simulink model "AcasXuClosedLoop_all"  (Simulink 10.0)
settings: Solver=VariableStepAuto  StartTime=0.0  StopTime=20  RelTol=1e-3
libraries: neural x14040, simulink x2
(part 35 of 534)

== system AcasXuClosedLoop_all/networks/Networks_m_8/AcasXu_4_8/Process Output 1 ==
  a : Inport  PortDimensions=5
      mask Display: e = 0.06;c1 = [120 230 180]/255;c2 = ([120 230 180]+0)/(256+128);c3 = ([120 230 180]+128)/(256+128);patch([0 1 1 0],[0 0 1 1],c1);patch([0 1 1 1-e 1-e 0+e],[0 0 1 1-e 0+e 0+e],c2);patch([0 0 1 1-e 0+e 0+e],[0 1 1 1-e 1-e 0+e],c3);plot([0 1 1 0 0],[0 0 1 1 0]);
  y : Outport  PortDimensions=5
      mask Display: e = 0.06;c1 = [120 230 180]/255;c2 = ([120 230 180]+0)/(256+128);c3 = ([120 230 180]+128)/(256+128);patch([0 1 1 0],[0 0 1 1],c1);patch([0 1 1 1-e 1-e 0+e],[0 0 1 1-e 0+e 0+e],c2);patch([0 0 1 1-e 0+e 0+e],[0 1 1 1-e 1-e 0+e],c3);plot([0 1 1 0 0],[0 0 1 1 0]);
  wires:
  a/1 -> y/1

== system AcasXuClosedLoop_all/networks/Networks_m_8/AcasXu_5_8/Layer 1 ==
  "p{1}" : Inport  PortDimensions=5
      mask Display: e = 0.06;c1 = [120 230 180]/255;c2 = ([120 230 180]+0)/(256+128);c3 = ([120 230 180]+128)/(256+128);patch([0 1 1 0],[0 0 1 1],c1);patch([0 1 1 1-e 1-e 0+e],[0 0 1 1-e 0+e 0+e],c2);patch([0 0 1 1-e 0+e 0+e],[0 1 1 1-e 1-e 0+e],c3);plot([0 1 1 0 0],[0 0 1 1 0]);
  Delays 1 : Subsystem
      mask Display: e = 0.06;c1 = [120 150 230]/255;c2 = ([120 150 230]+0)/383;c3 = ([120 150 230]+128)/383;patch([0 1 1 0],[0 0 1 1],c1);patch([0 1 1 1-e 1-e 0+e],[0 0 1 1-e 0+e 0+e],c2);patch([0 0 1 1-e 0+e 0+e],[0 1 1 1-e 1-e 0+e],c3);plot([0 1 1 0 0],[0 0 1 1 0]);disp('0');
  "IW{1,1}" : Subsystem
      mask Display: e = 0.06;c1 = [120 150 230]/255;c2 = ([120 150 230]+0)/383;c3 = ([120 150 230]+128)/383;patch([0 1 1 0],[0 0 1 1],c1);patch([0 1 1 1-e 1-e 0+e],[0 0 1 1-e 0+e 0+e],c2);patch([0 0 1 1-e 0+e 0+e],[0 1 1 1-e 1-e 0+e],c3);plot([0 1 1 0 0],[0 0 1 1 0]);disp('W');
  "b{1}" : Constant  Value=[-0.319583000000000005957900839348440058529376983642578125;-0.3150749999999999939603867460391484200954437255859375;-0.09534789999999999932089878029728424735367298126220703125;-0.155059000000000002383870878475136123597621917724609375;0.034337600000000002842259760882370756007730960845947265625;-0.421516000000000001790567694115452468395233154296875;0.0857165000000000010249578963339445181190967559814453125;-0.46167700000000000404298816647497005760669708251953125;-0.176536999999999999477751089216326363384723663330078125;-0.05784999999999999864552790995730902068316936492919921875;-0.0216248999999999989840571146260117529891431331634521484375;0.298148000000000024112267738018999807536602020263671875;-0.2051790000000000002700062395888380706310272216796875;-0.27237699999999998023980651851161383092403411865234375;-0.05032319999999999848849796535432687960565090179443359375;0.1063159999999999938413708377993316389620304107666015625;-0.144828000000000012281731187613331712782382965087890625;-0.33846900000000001984545860977959819138050079345703125;-0.0590398000000000033882230354720377363264560699462890625;0.0077789299999999998724842242836530203931033611297607421875;-0.2140189999999999870272660018599708564579486846923828125;-0.422790999999999972391862002041307277977466583251953125;0.2399779999999999968718356058161589317023754119873046875;-0.15526899999999999035793507573544047772884368896484375;-0.1191190000000000026592061885821749456226825714111328125;-0.489229999999999998205879592205747030675411224365234375;-0.01832330000000000069793060220035840757191181182861328125;-0.036199299999999996757527043200752814300358295440673828125;-0.42294500000000001538325022920616902410984039306640625;-0.62282000000000004025224598080967552959918975830078125;-0.05856619999999999881534762380397296510636806488037109375;0.13910400000000000542144107384956441819667816162109375;-0.310653999999999985703880156506784260272979736328125;0.0285514000000000010837108987971078022383153438568115234375;-0.341386000000000022769341967432410456240177154541015625;-0.038599500000000001864730592160412925295531749725341796875;0.11351899999999999490984947669858229346573352813720703125;-0.337141999999999997239541471572010777890682220458984375;-0.386535999999999990706101016257889568805694580078125;-0.328332000000000012729373111142194829881191253662109375;-0.1999869999999999981010745386811322532594203948974609375;-0.10244399999999999339994616320836939848959445953369140625;0.182161999999999990595966892215074039995670318603515625;-0.2535279999999999755999624539981596171855926513671875;-0.04500769999999999770867731285761692561209201812744140625;-0.07817380000000000161985980184908839873969554901123046875;-0.3433370000000000032969182939268648624420166015625;0.11499099999999999599520350557213532738387584686279296875;0.378197999999999978637532649372587911784648895263671875;-0.2001010000000000010889067425523535348474979400634765625]
      mask Display: e = 0.06;c1 = [120 150 230]/255;c2 = ([120 150 230]+0)/383;c3 = ([120 150 230]+128)/383;patch([0 1 1 0],[0 0 1 1],c1);patch([0 1 1 1-e 1-e 0+e],[0 0 1 1-e 0+e 0+e],c2);patch([0 0 1 1-e 0+e 0+e],[0 1 1 1-e 1-e 0+e],c3);plot([0 1 1 0 0],[0 0 1 1 0]);disp('b');
  netsum : Sum  Inputs=2  SaturateOnIntegerOverflow=on  InputSameDT=on  OutDataTypeStr=Inherit: Same as first input
      mask Display: e = 0.06;c1 = [120 150 230]/255;c2 = ([120 150 230]+0)/383;c3 = ([120 150 230]+128)/383;patch([0 1 1 0],[0 0 1 1],c1);patch([0 1 1 1-e 1-e 0+e],[0 0 1 1-e 0+e 0+e],c2);patch([0 0 1 1-e 0+e 0+e],[0 1 1 1-e 1-e 0+e],c3);plot([0 1 1 0 0],[0 0 1 1 0]);disp('+');
  poslin : neural/Transfer Functions/poslin
      settings: SourceProductName=Deep Learning Toolbox  SourceProductBaseCode=NN
  "a{1}" : Outport  InitialOutput=[0;0;0;0;0;0;0;0;0;0;0;0;0;0;0;0;0;0;0;0;0;0;0;0;0;0;0;0;0;0;0;0;0;0;0;0;0;0;0;0;0;0;0;0;0;0;0;0;0;0]
      mask Display: e = 0.06;c1 = [120 230 180]/255;c2 = ([120 230 180]+0)/(256+128);c3 = ([120 230 180]+128)/(256+128);patch([0 1 1 0],[0 0 1 1],c1);patch([0 1 1 1-e 1-e 0+e],[0 0 1 1-e 0+e 0+e],c2);patch([0 0 1 1-e 0+e 0+e],[0 1 1 1-e 1-e 0+e],c3);plot([0 1 1 0 0],[0 0 1 1 0]);
  wires:
  poslin/1 -> "a{1}"/1
  netsum/1 -> poslin/1
  "b{1}"/1 -> netsum/2
  "IW{1,1}"/1("iz{1,1}") -> netsum/1
  Delays 1/1("pd{1,1}") -> "IW{1,1}"/1("pd{1,1}")
  "p{1}"/1 -> Delays 1/1("p{1}")

== system AcasXuClosedLoop_all/networks/Networks_m_8/AcasXu_5_8/Layer 2 ==
  "a{1} " : Inport  PortDimensions=50
      mask Display: e = 0.06;c1 = [120 230 180]/255;c2 = ([120 230 180]+0)/(256+128);c3 = ([120 230 180]+128)/(256+128);patch([0 1 1 0],[0 0 1 1],c1);patch([0 1 1 1-e 1-e 0+e],[0 0 1 1-e 0+e 0+e],c2);patch([0 0 1 1-e 0+e 0+e],[0 1 1 1-e 1-e 0+e],c3);plot([0 1 1 0 0],[0 0 1 1 0]);
  Delays 1 : Subsystem
      mask Display: e = 0.06;c1 = [120 150 230]/255;c2 = ([120 150 230]+0)/383;c3 = ([120 150 230]+128)/383;patch([0 1 1 0],[0 0 1 1],c1);patch([0 1 1 1-e 1-e 0+e],[0 0 1 1-e 0+e 0+e],c2);patch([0 0 1 1-e 0+e 0+e],[0 1 1 1-e 1-e 0+e],c3);plot([0 1 1 0 0],[0 0 1 1 0]);disp('0');
  "LW{2,1}" : Subsystem
      mask Display: e = 0.06;c1 = [120 150 230]/255;c2 = ([120 150 230]+0)/383;c3 = ([120 150 230]+128)/383;patch([0 1 1 0],[0 0 1 1],c1);patch([0 1 1 1-e 1-e 0+e],[0 0 1 1-e 0+e 0+e],c2);patch([0 0 1 1-e 0+e 0+e],[0 1 1 1-e 1-e 0+e],c3);plot([0 1 1 0 0],[0 0 1 1 0]);disp('W');
  "b{2}" : Constant  Value=[0.8293500000000000316191517413244582712650299072265625;-0.037375499999999999001243367047209176234900951385498046875;-1.9736100000000000864730509420041926205158233642578125;-1.100190000000000001278976924368180334568023681640625;-1.525160000000000071196382123162038624286651611328125;-0.92608100000000004303757350498926825821399688720703125;-2.50565000000000015489831639570184051990509033203125;-0.023995200000000001250288761411866289563477039337158203125;0.22440599999999999436539610542240552604198455810546875;0.22149499999999999744204615126363933086395263671875;0.244974999999999998312461002569762058556079864501953125;0.685756000000000032201796784647740423679351806640625;-0.1502779999999999949178430824758834205567836761474609375;0.7911420000000000118944853966240771114826202392578125;-1.4556899999999999284483465089579112827777862548828125;-0.75029800000000002047073621724848635494709014892578125;-0.0119462000000000005683897796870951424352824687957763671875;-0.93398700000000001164579543910804204642772674560546875;0.333909999999999984598986202399828471243381500244140625;-0.00553113000000000042122305643488289206288754940032958984375;0.54637299999999999755573298898525536060333251953125;-0.0108263000000000005063061081500563886947929859161376953125;1.1451299999999999812416717759333550930023193359375;1.1682799999999999851496568226139061152935028076171875;1.466369999999999951256768326857127249240875244140625;-0.0118300999999999996437072269372947630472481250762939453125;0.449658999999999975383246919591329060494899749755859375;0.82613000000000003097966327914036810398101806640625;0.0337432000000000009709566484161769039928913116455078125;-0.220237999999999989331200822562095709145069122314453125;-0.600424999999999986499688020558096468448638916015625;-0.76253300000000001634958834983990527689456939697265625;-0.1156770000000000020445867221496882848441600799560546875;0.0762764999999999970814457128653884865343570709228515625;0.54749499999999995392130358595750294625759124755859375;-1.4459299999999999375432935266871936619281768798828125;-0.437894999999999978701481495590996928513050079345703125;0.1760150000000000047872816821836750023066997528076171875;-0.40112599999999998257038669180474244058132171630859375;-0.01016309999999999956477036988644613302312791347503662109375;0.044338099999999998346300600360336829908192157745361328125;-0.005221739999999999888580237694668539916165173053741455078125;1.54872999999999994003019310184754431247711181640625;-1.30051000000000005485389920067973434925079345703125;-0.94384800000000002029310053330846130847930908203125;-0.85577000000000003065991904804832302033901214599609375;-0.030165100000000000246558329308754764497280120849609375;-0.289264000000000021106671965753776021301746368408203125;-0.00971820999999999969587438641838161856867372989654541015625;0.41300999999999998824051772317034192383289337158203125]
      mask Display: e = 0.06;c1 = [120 150 230]/255;c2 = ([120 150 230]+0)/383;c3 = ([120 150 230]+128)/383;patch([0 1 1 0],[0 0 1 1],c1);patch([0 1 1 1-e 1-e 0+e],[0 0 1 1-e 0+e 0+e],c2);patch([0 0 1 1-e 0+e 0+e],[0 1 1 1-e 1-e 0+e],c3);plot([0 1 1 0 0],[0 0 1 1 0]);disp('b');
  netsum : Sum  Inputs=2  SaturateOnIntegerOverflow=on  InputSameDT=on  OutDataTypeStr=Inherit: Same as first input
      mask Display: e = 0.06;c1 = [120 150 230]/255;c2 = ([120 150 230]+0)/383;c3 = ([120 150 230]+128)/383;patch([0 1 1 0],[0 0 1 1],c1);patch([0 1 1 1-e 1-e 0+e],[0 0 1 1-e 0+e 0+e],c2);patch([0 0 1 1-e 0+e 0+e],[0 1 1 1-e 1-e 0+e],c3);plot([0 1 1 0 0],[0 0 1 1 0]);disp('+');
  poslin : neural/Transfer Functions/poslin
      settings: SourceProductName=Deep Learning Toolbox  SourceProductBaseCode=NN
  "a{2}" : Outport  InitialOutput=[0;0;0;0;0;0;0;0;0;0;0;0;0;0;0;0;0;0;0;0;0;0;0;0;0;0;0;0;0;0;0;0;0;0;0;0;0;0;0;0;0;0;0;0;0;0;0;0;0;0]
      mask Display: e = 0.06;c1 = [120 230 180]/255;c2 = ([120 230 180]+0)/(256+128);c3 = ([120 230 180]+128)/(256+128);patch([0 1 1 0],[0 0 1 1],c1);patch([0 1 1 1-e 1-e 0+e],[0 0 1 1-e 0+e 0+e],c2);patch([0 0 1 1-e 0+e 0+e],[0 1 1 1-e 1-e 0+e],c3);plot([0 1 1 0 0],[0 0 1 1 0]);
  wires:
  poslin/1 -> "a{2}"/1
  netsum/1 -> poslin/1
  "b{2}"/1 -> netsum/2
  "LW{2,1}"/1("lz{2,1}") -> netsum/1
  Delays 1/1("pd{2,1}") -> "LW{2,1}"/1("ad{2,1}")
  "a{1} "/1 -> Delays 1/1("p{2}")

== system AcasXuClosedLoop_all/networks/Networks_m_8/AcasXu_5_8/Layer 3 ==
  "a{2} " : Inport  PortDimensions=50
      mask Display: e = 0.06;c1 = [120 230 180]/255;c2 = ([120 230 180]+0)/(256+128);c3 = ([120 230 180]+128)/(256+128);patch([0 1 1 0],[0 0 1 1],c1);patch([0 1 1 1-e 1-e 0+e],[0 0 1 1-e 0+e 0+e],c2);patch([0 0 1 1-e 0+e 0+e],[0 1 1 1-e 1-e 0+e],c3);plot([0 1 1 0 0],[0 0 1 1 0]);
  Delays 1 : Subsystem
      mask Display: e = 0.06;c1 = [120 150 230]/255;c2 = ([120 150 230]+0)/383;c3 = ([120 150 230]+128)/383;patch([0 1 1 0],[0 0 1 1],c1);patch([0 1 1 1-e 1-e 0+e],[0 0 1 1-e 0+e 0+e],c2);patch([0 0 1 1-e 0+e 0+e],[0 1 1 1-e 1-e 0+e],c3);plot([0 1 1 0 0],[0 0 1 1 0]);disp('0');
  "LW{3,2}" : Subsystem
      mask Display: e = 0.06;c1 = [120 150 230]/255;c2 = ([120 150 230]+0)/383;c3 = ([120 150 230]+128)/383;patch([0 1 1 0],[0 0 1 1],c1);patch([0 1 1 1-e 1-e 0+e],[0 0 1 1-e 0+e 0+e],c2);patch([0 0 1 1-e 0+e 0+e],[0 1 1 1-e 1-e 0+e],c3);plot([0 1 1 0 0],[0 0 1 1 0]);disp('W');
  "b{3}" : Constant  Value=[0.6144659999999999566711039733490906655788421630859375;-1.150679999999999925108795650885440409183502197265625;1.506089999999999928803617876837961375713348388671875;-0.0218403999999999993975041689964200486429035663604736328125;1.099439999999999972857267493964172899723052978515625;0.65309399999999995234389871257008053362369537353515625;-0.67926399999999997891819702999782748520374298095703125;-0.586089999999999999857891452847979962825775146484375;0.3059439999999999937330130705959163606166839599609375;-0.9985239999999999671587147531681694090366363525390625;-1.3036700000000001065103560904390178620815277099609375;1.218690000000000051016968427575193345546722412109375;-1.231030000000000068638428274425677955150604248046875;-0.269017000000000006121325668573263101279735565185546875;-0.1322319999999999884376933323437697254121303558349609375;-0.64689399999999996904165300293243490159511566162109375;-1.992599999999999926814098216709680855274200439453125;-0.93583600000000000118660636871936731040477752685546875;-2.670059999999999877928757996414788067340850830078125;0.6867689999999999628244040650315582752227783203125;-1.8478300000000000835598257253877818584442138671875;-0.878910999999999997811528373858891427516937255859375;-1.1449800000000001087840928448713384568691253662109375;-0.644249999999999989341858963598497211933135986328125;-0.55706800000000000760991269999067299067974090576171875;-0.145936000000000010157208407690632157027721405029296875;-2.324780000000000068638428274425677955150604248046875;1.6987200000000000077449158197850920259952545166015625;-0.03332290000000000251834109121773508377373218536376953125;0.91283700000000000951416723182774148881435394287109375;-0.149413999999999991263877063829568214714527130126953125;-2.640660000000000007247535904753021895885467529296875;-0.0249848000000000014519940805257647298276424407958984375;-0.76798299999999997123012462907354347407817840576171875;1.3071900000000000741096073397784493863582611083984375;-1.425289999999999945856643535080365836620330810546875;-0.12238300000000000566924285294589935801923274993896484375;-0.01368040000000000054602988797114448971115052700042724609375;-0.7686349999999999571542730336659587919712066650390625;-0.1422120000000000050732751333271153271198272705078125;-0.176196999999999992514432278767344541847705841064453125;-1.058829999999999937898564894567243754863739013671875;-1.5299400000000000776623210185789503157138824462890625;-0.736450000000000049027448767446912825107574462890625;-0.84162999999999998923527755323448218405246734619140625;-0.043412899999999997324717782021252787671983242034912109375;-1.323560000000000069775296651641838252544403076171875;1.27156000000000002359001882723532617092132568359375;-1.148660000000000014352963262354023754596710205078125;-1.66657000000000010686562745831906795501708984375]
      mask Display: e = 0.06;c1 = [120 150 230]/255;c2 = ([120 150 230]+0)/383;c3 = ([120 150 230]+128)/383;patch([0 1 1 0],[0 0 1 1],c1);patch([0 1 1 1-e 1-e 0+e],[0 0 1 1-e 0+e 0+e],c2);patch([0 0 1 1-e 0+e 0+e],[0 1 1 1-e 1-e 0+e],c3);plot([0 1 1 0 0],[0 0 1 1 0]);disp('b');
  netsum : Sum  Inputs=2  SaturateOnIntegerOverflow=on  InputSameDT=on  OutDataTypeStr=Inherit: Same as first input
      mask Display: e = 0.06;c1 = [120 150 230]/255;c2 = ([120 150 230]+0)/383;c3 = ([120 150 230]+128)/383;patch([0 1 1 0],[0 0 1 1],c1);patch([0 1 1 1-e 1-e 0+e],[0 0 1 1-e 0+e 0+e],c2);patch([0 0 1 1-e 0+e 0+e],[0 1 1 1-e 1-e 0+e],c3);plot([0 1 1 0 0],[0 0 1 1 0]);disp('+');
  poslin : neural/Transfer Functions/poslin
      settings: SourceProductName=Deep Learning Toolbox  SourceProductBaseCode=NN
  "a{3}" : Outport  InitialOutput=[0;0;0;0;0;0;0;0;0;0;0;0;0;0;0;0;0;0;0;0;0;0;0;0;0;0;0;0;0;0;0;0;0;0;0;0;0;0;0;0;0;0;0;0;0;0;0;0;0;0]
      mask Display: e = 0.06;c1 = [120 230 180]/255;c2 = ([120 230 180]+0)/(256+128);c3 = ([120 230 180]+128)/(256+128);patch([0 1 1 0],[0 0 1 1],c1);patch([0 1 1 1-e 1-e 0+e],[0 0 1 1-e 0+e 0+e],c2);patch([0 0 1 1-e 0+e 0+e],[0 1 1 1-e 1-e 0+e],c3);plot([0 1 1 0 0],[0 0 1 1 0]);
  wires:
  poslin/1 -> "a{3}"/1
  netsum/1 -> poslin/1
  "b{3}"/1 -> netsum/2
  "LW{3,2}"/1("lz{3,2}") -> netsum/1
  Delays 1/1("pd{3,2}") -> "LW{3,2}"/1("ad{3,2}")
  "a{2} "/1 -> Delays 1/1("p{3}")

== system AcasXuClosedLoop_all/networks/Networks_m_8/AcasXu_5_8/Layer 4 ==
  "a{3} " : Inport  PortDimensions=50
      mask Display: e = 0.06;c1 = [120 230 180]/255;c2 = ([120 230 180]+0)/(256+128);c3 = ([120 230 180]+128)/(256+128);patch([0 1 1 0],[0 0 1 1],c1);patch([0 1 1 1-e 1-e 0+e],[0 0 1 1-e 0+e 0+e],c2);patch([0 0 1 1-e 0+e 0+e],[0 1 1 1-e 1-e 0+e],c3);plot([0 1 1 0 0],[0 0 1 1 0]);
  Delays 1 : Subsystem
      mask Display: e = 0.06;c1 = [120 150 230]/255;c2 = ([120 150 230]+0)/383;c3 = ([120 150 230]+128)/383;patch([0 1 1 0],[0 0 1 1],c1);patch([0 1 1 1-e 1-e 0+e],[0 0 1 1-e 0+e 0+e],c2);patch([0 0 1 1-e 0+e 0+e],[0 1 1 1-e 1-e 0+e],c3);plot([0 1 1 0 0],[0 0 1 1 0]);disp('0');
  "LW{4,3}" : Subsystem
      mask Display: e = 0.06;c1 = [120 150 230]/255;c2 = ([120 150 230]+0)/383;c3 = ([120 150 230]+128)/383;patch([0 1 1 0],[0 0 1 1],c1);patch([0 1 1 1-e 1-e 0+e],[0 0 1 1-e 0+e 0+e],c2);patch([0 0 1 1-e 0+e 0+e],[0 1 1 1-e 1-e 0+e],c3);plot([0 1 1 0 0],[0 0 1 1 0]);disp('W');
  "b{4}" : Constant  Value=[0.702555999999999958305352265597321093082427978515625;1.3879099999999999770494696349487639963626861572265625;0.3384570000000000078443918027915060520172119140625;1.8266199999999999104005610206513665616512298583984375;0.90297499999999997211119762141606770455837249755859375;-1.5656499999999999861444166526780463755130767822265625;2.740489999999999870539113544509746134281158447265625;-2.53134000000000014551915228366851806640625;-1.0149699999999999278088580467738211154937744140625;-0.41894500000000001183053655040566809475421905517578125;1.289029999999999898108171692001633346080780029296875;-1.0280400000000000648725517748971469700336456298828125;4.997939999999999827196006663143634796142578125;0.81166700000000002734878989940625615417957305908203125;-1.834379999999999899529257163521833717823028564453125;2.332240000000000090807361630140803754329681396484375;0.12376600000000000101341157687784289009869098663330078125;-0.2025119999999999975681674868610571138560771942138671875;1.195019999999999971151964928139932453632354736328125;0.8384679999999999910897940935683436691761016845703125;0.641885999999999956600049699773080646991729736328125;0.007645029999999999707760434120018544490449130535125732421875;-2.253229999999999844106923774234019219875335693359375;0.70936600000000005206146624914254061877727508544921875;-0.039787999999999996980637462229424272663891315460205078125;0.768780000000000018900436771218664944171905517578125;-0.97767099999999995674215824692510068416595458984375;0.211146000000000000351718654201249592006206512451171875;-0.83741500000000002046363078989088535308837890625;-1.753509999999999902087211012258194386959075927734375;0.397567999999999976967757220336352474987506866455078125;-0.417839000000000015955237131493049673736095428466796875;1.2331399999999999028688080215943045914173126220703125;1.6854999999999999982236431605997495353221893310546875;0.06742479999999999307203069065508316271007061004638671875;2.401850000000000040500935938325710594654083251953125;-0.0266778000000000015123902130653732456266880035400390625;-2.4404599999999998516386767732910811901092529296875;0.26406600000000002292921408297843299806118011474609375;-0.6424499999999999655386773156351409852504730224609375;1.0294600000000000417088585891178809106349945068359375;0.84932700000000005413625103756203316152095794677734375;0.93856700000000004013855914308805949985980987548828125;1.319269999999999942730255497735925018787384033203125;-1.5707999999999999740651901447563432157039642333984375;0.8400069999999999481588019989430904388427734375;0.8738289999999999668744976588641293346881866455078125;-0.1663550000000000028688162956314045004546642303466796875;1.4832799999999999318589516406063921749591827392578125;0.98826300000000000256505927609396167099475860595703125]
      mask Display: e = 0.06;c1 = [120 150 230]/255;c2 = ([120 150 230]+0)/383;c3 = ([120 150 230]+128)/383;patch([0 1 1 0],[0 0 1 1],c1);patch([0 1 1 1-e 1-e 0+e],[0 0 1 1-e 0+e 0+e],c2);patch([0 0 1 1-e 0+e 0+e],[0 1 1 1-e 1-e 0+e],c3);plot([0 1 1 0 0],[0 0 1 1 0]);disp('b');
  netsum : Sum  Inputs=2  SaturateOnIntegerOverflow=on  InputSameDT=on  OutDataTypeStr=Inherit: Same as first input
      mask Display: e = 0.06;c1 = [120 150 230]/255;c2 = ([120 150 230]+0)/383;c3 = ([120 150 230]+128)/383;patch([0 1 1 0],[0 0 1 1],c1);patch([0 1 1 1-e 1-e 0+e],[0 0 1 1-e 0+e 0+e],c2);patch([0 0 1 1-e 0+e 0+e],[0 1 1 1-e 1-e 0+e],c3);plot([0 1 1 0 0],[0 0 1 1 0]);disp('+');
  poslin : neural/Transfer Functions/poslin
      settings: SourceProductName=Deep Learning Toolbox  SourceProductBaseCode=NN
  "a{4}" : Outport  InitialOutput=[0;0;0;0;0;0;0;0;0;0;0;0;0;0;0;0;0;0;0;0;0;0;0;0;0;0;0;0;0;0;0;0;0;0;0;0;0;0;0;0;0;0;0;0;0;0;0;0;0;0]
      mask Display: e = 0.06;c1 = [120 230 180]/255;c2 = ([120 230 180]+0)/(256+128);c3 = ([120 230 180]+128)/(256+128);patch([0 1 1 0],[0 0 1 1],c1);patch([0 1 1 1-e 1-e 0+e],[0 0 1 1-e 0+e 0+e],c2);patch([0 0 1 1-e 0+e 0+e],[0 1 1 1-e 1-e 0+e],c3);plot([0 1 1 0 0],[0 0 1 1 0]);
  wires:
  poslin/1 -> "a{4}"/1
  netsum/1 -> poslin/1
  "b{4}"/1 -> netsum/2
  "LW{4,3}"/1("lz{4,3}") -> netsum/1
  Delays 1/1("pd{4,3}") -> "LW{4,3}"/1("ad{4,3}")
  "a{3} "/1 -> Delays 1/1("p{4}")

== system AcasXuClosedLoop_all/networks/Networks_m_8/AcasXu_5_8/Layer 5 ==
  "a{4} " : Inport  PortDimensions=50
      mask Display: e = 0.06;c1 = [120 230 180]/255;c2 = ([120 230 180]+0)/(256+128);c3 = ([120 230 180]+128)/(256+128);patch([0 1 1 0],[0 0 1 1],c1);patch([0 1 1 1-e 1-e 0+e],[0 0 1 1-e 0+e 0+e],c2);patch([0 0 1 1-e 0+e 0+e],[0 1 1 1-e 1-e 0+e],c3);plot([0 1 1 0 0],[0 0 1 1 0]);
  Delays 1 : Subsystem
      mask Display: e = 0.06;c1 = [120 150 230]/255;c2 = ([120 150 230]+0)/383;c3 = ([120 150 230]+128)/383;patch([0 1 1 0],[0 0 1 1],c1);patch([0 1 1 1-e 1-e 0+e],[0 0 1 1-e 0+e 0+e],c2);patch([0 0 1 1-e 0+e 0+e],[0 1 1 1-e 1-e 0+e],c3);plot([0 1 1 0 0],[0 0 1 1 0]);disp('0');
  "LW{5,4}" : Subsystem
      mask Display: e = 0.06;c1 = [120 150 230]/255;c2 = ([120 150 230]+0)/383;c3 = ([120 150 230]+128)/383;patch([0 1 1 0],[0 0 1 1],c1);patch([0 1 1 1-e 1-e 0+e],[0 0 1 1-e 0+e 0+e],c2);patch([0 0 1 1-e 0+e 0+e],[0 1 1 1-e 1-e 0+e],c3);plot([0 1 1 0 0],[0 0 1 1 0]);disp('W');
  "b{5}" : Constant  Value=[-3.1370599999999999596411726088263094425201416015625;-1.6457100000000000061817218011128716170787811279296875;-1.575390000000000068069994085817597806453704833984375;-0.271681000000000005822897719554021023213863372802734375;0.4875220000000000108997255665599368512630462646484375;1.5458700000000000773781039242749102413654327392578125;-1.087099999999999955235807647113688290119171142578125;-0.096647800000000005926636958974995650351047515869140625;1.4024900000000000144240175359300337731838226318359375;0.8425219999999999931361571725574322044849395751953125;0.6656800000000000494537744089029729366302490234375;-1.018520000000000091944230007356964051723480224609375;1.1327899999999999636202119290828704833984375;2.48627000000000020207835405017249286174774169921875;0.6509570000000000078443918027915060520172119140625;-0.042899100000000002508304675075123668648302555084228515625;-1.2160800000000000498090457767830230295658111572265625;-2.937650000000000094502183856093324720859527587890625;1.667829999999999923687710179365240037441253662109375;-3.67757999999999984908072292455472052097320556640625;0.6318070000000000074891204349114559590816497802734375;-0.479887000000000008004263918337528593838214874267578125;2.622430000000000038795633372501470148563385009765625;4.37605000000000021742607714259065687656402587890625;0.48666500000000001424638185199000872671604156494140625;-2.939290000000000180335746335913427174091339111328125;-0.4961160000000000014352963262354023754596710205078125;2.286119999999999929940486254054121673107147216796875;-1.5830999999999999516830939683131873607635498046875;-1.6355999999999999428013097713119350373744964599609375;-2.411880000000000023874235921539366245269775390625;-1.1924799999999999844391140868538059294223785400390625;-2.653000000000000024868995751603506505489349365234375;0.68499900000000002453504066579625941812992095947265625;0.9526369999999999560458263658802025020122528076171875;-1.234669999999999934203742668614722788333892822265625;2.191619999999999901518776823650114238262176513671875;-4.706559999999999632791514159180223941802978515625;-0.475185000000000024034108037085388787090778350830078125;0.324878999999999973358200122675043530762195587158203125;1.14918999999999993377741702715866267681121826171875;-0.56789500000000003865352482534945011138916015625;-3.545409999999999950404117043945007026195526123046875;1.9624900000000000677147227179375477135181427001953125;-0.057324199999999998766941899930316139943897724151611328125;-1.4606600000000000694200252837617881596088409423828125;1.7740199999999999302957576219341717660427093505859375;0.11874200000000000032596148002994596026837825775146484375;-1.5381000000000000227373675443232059478759765625;-4.32064000000000003609557097661308944225311279296875]
      mask Display: e = 0.06;c1 = [120 150 230]/255;c2 = ([120 150 230]+0)/383;c3 = ([120 150 230]+128)/383;patch([0 1 1 0],[0 0 1 1],c1);patch([0 1 1 1-e 1-e 0+e],[0 0 1 1-e 0+e 0+e],c2);patch([0 0 1 1-e 0+e 0+e],[0 1 1 1-e 1-e 0+e],c3);plot([0 1 1 0 0],[0 0 1 1 0]);disp('b');
  netsum : Sum  Inputs=2  SaturateOnIntegerOverflow=on  InputSameDT=on  OutDataTypeStr=Inherit: Same as first input
      mask Display: e = 0.06;c1 = [120 150 230]/255;c2 = ([120 150 230]+0)/383;c3 = ([120 150 230]+128)/383;patch([0 1 1 0],[0 0 1 1],c1);patch([0 1 1 1-e 1-e 0+e],[0 0 1 1-e 0+e 0+e],c2);patch([0 0 1 1-e 0+e 0+e],[0 1 1 1-e 1-e 0+e],c3);plot([0 1 1 0 0],[0 0 1 1 0]);disp('+');
  poslin : neural/Transfer Functions/poslin
      settings: SourceProductName=Deep Learning Toolbox  SourceProductBaseCode=NN
  "a{5}" : Outport  InitialOutput=[0;0;0;0;0;0;0;0;0;0;0;0;0;0;0;0;0;0;0;0;0;0;0;0;0;0;0;0;0;0;0;0;0;0;0;0;0;0;0;0;0;0;0;0;0;0;0;0;0;0]
      mask Display: e = 0.06;c1 = [120 230 180]/255;c2 = ([120 230 180]+0)/(256+128);c3 = ([120 230 180]+128)/(256+128);patch([0 1 1 0],[0 0 1 1],c1);patch([0 1 1 1-e 1-e 0+e],[0 0 1 1-e 0+e 0+e],c2);patch([0 0 1 1-e 0+e 0+e],[0 1 1 1-e 1-e 0+e],c3);plot([0 1 1 0 0],[0 0 1 1 0]);
  wires:
  poslin/1 -> "a{5}"/1
  netsum/1 -> poslin/1
  "b{5}"/1 -> netsum/2
  "LW{5,4}"/1("lz{5,4}") -> netsum/1
  Delays 1/1("pd{5,4}") -> "LW{5,4}"/1("ad{5,4}")
  "a{4} "/1 -> Delays 1/1("p{5}")

== system AcasXuClosedLoop_all/networks/Networks_m_8/AcasXu_5_8/Layer 6 ==
  "a{5} " : Inport  PortDimensions=50
      mask Display: e = 0.06;c1 = [120 230 180]/255;c2 = ([120 230 180]+0)/(256+128);c3 = ([120 230 180]+128)/(256+128);patch([0 1 1 0],[0 0 1 1],c1);patch([0 1 1 1-e 1-e 0+e],[0 0 1 1-e 0+e 0+e],c2);patch([0 0 1 1-e 0+e 0+e],[0 1 1 1-e 1-e 0+e],c3);plot([0 1 1 0 0],[0 0 1 1 0]);
  Delays 1 : Subsystem
      mask Display: e = 0.06;c1 = [120 150 230]/255;c2 = ([120 150 230]+0)/383;c3 = ([120 150 230]+128)/383;patch([0 1 1 0],[0 0 1 1],c1);patch([0 1 1 1-e 1-e 0+e],[0 0 1 1-e 0+e 0+e],c2);patch([0 0 1 1-e 0+e 0+e],[0 1 1 1-e 1-e 0+e],c3);plot([0 1 1 0 0],[0 0 1 1 0]);disp('0');
  "LW{6,5}" : Subsystem
      mask Display: e = 0.06;c1 = [120 150 230]/255;c2 = ([120 150 230]+0)/383;c3 = ([120 150 230]+128)/383;patch([0 1 1 0],[0 0 1 1],c1);patch([0 1 1 1-e 1-e 0+e],[0 0 1 1-e 0+e 0+e],c2);patch([0 0 1 1-e 0+e 0+e],[0 1 1 1-e 1-e 0+e],c3);plot([0 1 1 0 0],[0 0 1 1 0]);disp('W');
  "b{6}" : Constant  Value=[0.5970990000000000463842297904193401336669921875;3.27104999999999979110043568653054535388946533203125;-0.73021999999999998021138480908120982348918914794921875;5.6033600000000003404920789762400090694427490234375;0.058062099999999998434230263910649227909743785858154296875;-3.54084999999999983089082888909615576267242431640625;3.0053000000000000824229573481716215610504150390625;-0.033653700000000001668620797090625273995101451873779296875;-0.4597029999999999727577915109577588737010955810546875;-3.43447000000000013386625141720287501811981201171875;1.75800000000000000710542735760100185871124267578125;0.76190199999999996816058001058991067111492156982421875;-3.917429999999999967741359796491451561450958251953125;1.390589999999999992752464095246978104114532470703125;1.1082199999999999828759200681815855205059051513671875;-1.5958600000000000562749846721999347209930419921875;-0.19688600000000000544986278327996842563152313232421875;-2.475429999999999797211103214067406952381134033203125;-0.1586950000000000027267077484793844632804393768310546875;-2.147619999999999862438926356844604015350341796875;2.91274999999999995026200849679298698902130126953125;-2.2351299999999998391331246239133179187774658203125;-4.30694999999999961204366627498529851436614990234375;-0.029325400000000001465849663873086683452129364013671875;-0.014737699999999999411759432632607058621942996978759765625;-4.529379999999999739657141617499291896820068359375;0.06399839999999999695656782705555087886750698089599609375;4.03953999999999968650854498264379799365997314453125;0.59581600000000001227107304657693020999431610107421875;2.958439999999999958646412778762169182300567626953125;0.3424490000000000033963942769332788884639739990234375;1.580410000000000092512664195965044200420379638671875;0.45372299999999998743049900440382771193981170654296875;1.2287500000000000088817841970012523233890533447265625;0.70787400000000000321875859299325384199619293212890625;0.439097999999999988318677424103952944278717041015625;7.138700000000000045474735088646411895751953125;-1.1250199999999999089794755491311661899089813232421875;-2.954860000000000042064129956997931003570556640625;-1.0480899999999999661071115042432211339473724365234375;-2.107110000000000038511416278197430074214935302734375;1.720429999999999903792513578082434833049774169921875;-1.3264299999999999979394260662957094609737396240234375;0.85650400000000004308020606913487426936626434326171875;0.489951000000000025380586521350778639316558837890625;-1.5742700000000000581934500587522052228450775146484375;1.25326000000000004064304448547773063182830810546875;-3.311179999999999790105675856466405093669891357421875;1.8854699999999999793232063893810845911502838134765625;-0.8230049999999999865707422941341064870357513427734375]
      mask Display: e = 0.06;c1 = [120 150 230]/255;c2 = ([120 150 230]+0)/383;c3 = ([120 150 230]+128)/383;patch([0 1 1 0],[0 0 1 1],c1);patch([0 1 1 1-e 1-e 0+e],[0 0 1 1-e 0+e 0+e],c2);patch([0 0 1 1-e 0+e 0+e],[0 1 1 1-e 1-e 0+e],c3);plot([0 1 1 0 0],[0 0 1 1 0]);disp('b');
  netsum : Sum  Inputs=2  SaturateOnIntegerOverflow=on  InputSameDT=on  OutDataTypeStr=Inherit: Same as first input
      mask Display: e = 0.06;c1 = [120 150 230]/255;c2 = ([120 150 230]+0)/383;c3 = ([120 150 230]+128)/383;patch([0 1 1 0],[0 0 1 1],c1);patch([0 1 1 1-e 1-e 0+e],[0 0 1 1-e 0+e 0+e],c2);patch([0 0 1 1-e 0+e 0+e],[0 1 1 1-e 1-e 0+e],c3);plot([0 1 1 0 0],[0 0 1 1 0]);disp('+');
  poslin : neural/Transfer Functions/poslin
      settings: SourceProductName=Deep Learning Toolbox  SourceProductBaseCode=NN
  "a{6}" : Outport  InitialOutput=[0;0;0;0;0;0;0;0;0;0;0;0;0;0;0;0;0;0;0;0;0;0;0;0;0;0;0;0;0;0;0;0;0;0;0;0;0;0;0;0;0;0;0;0;0;0;0;0;0;0]
      mask Display: e = 0.06;c1 = [120 230 180]/255;c2 = ([120 230 180]+0)/(256+128);c3 = ([120 230 180]+128)/(256+128);patch([0 1 1 0],[0 0 1 1],c1);patch([0 1 1 1-e 1-e 0+e],[0 0 1 1-e 0+e 0+e],c2);patch([0 0 1 1-e 0+e 0+e],[0 1 1 1-e 1-e 0+e],c3);plot([0 1 1 0 0],[0 0 1 1 0]);
  wires:
  poslin/1 -> "a{6}"/1
  netsum/1 -> poslin/1
  "b{6}"/1 -> netsum/2
  "LW{6,5}"/1("lz{6,5}") -> netsum/1
  Delays 1/1("pd{6,5}") -> "LW{6,5}"/1("ad{6,5}")
  "a{5} "/1 -> Delays 1/1("p{6}")

== system AcasXuClosedLoop_all/networks/Networks_m_8/AcasXu_5_8/Layer 7 ==
  "a{6} " : Inport  PortDimensions=50
      mask Display: e = 0.06;c1 = [120 230 180]/255;c2 = ([120 230 180]+0)/(256+128);c3 = ([120 230 180]+128)/(256+128);patch([0 1 1 0],[0 0 1 1],c1);patch([0 1 1 1-e 1-e 0+e],[0 0 1 1-e 0+e 0+e],c2);patch([0 0 1 1-e 0+e 0+e],[0 1 1 1-e 1-e 0+e],c3);plot([0 1 1 0 0],[0 0 1 1 0]);
  Delays 1 : Subsystem
      mask Display: e = 0.06;c1 = [120 150 230]/255;c2 = ([120 150 230]+0)/383;c3 = ([120 150 230]+128)/383;patch([0 1 1 0],[0 0 1 1],c1);patch([0 1 1 1-e 1-e 0+e],[0 0 1 1-e 0+e 0+e],c2);patch([0 0 1 1-e 0+e 0+e],[0 1 1 1-e 1-e 0+e],c3);plot([0 1 1 0 0],[0 0 1 1 0]);disp('0');
  "LW{7,6}" : Subsystem
      mask Display: e = 0.06;c1 = [120 150 230]/255;c2 = ([120 150 230]+0)/383;c3 = ([120 150 230]+128)/383;patch([0 1 1 0],[0 0 1 1],c1);patch([0 1 1 1-e 1-e 0+e],[0 0 1 1-e 0+e 0+e],c2);patch([0 0 1 1-e 0+e 0+e],[0 1 1 1-e 1-e 0+e],c3);plot([0 1 1 0 0],[0 0 1 1 0]);disp('W');
  "b{7}" : Constant  Value=[-0.0200872999999999989062526850602807826362550258636474609375;0.018134799999999999531308247924243914894759654998779296875;-0.0185214999999999997692956554828924708999693393707275390625;0.0184039000000000008139267038131947629153728485107421875;-0.018308600000000001262190352235847967676818370819091796875]
      mask Display: e = 0.06;c1 = [120 150 230]/255;c2 = ([120 150 230]+0)/383;c3 = ([120 150 230]+128)/383;patch([0 1 1 0],[0 0 1 1],c1);patch([0 1 1 1-e 1-e 0+e],[0 0 1 1-e 0+e 0+e],c2);patch([0 0 1 1-e 0+e 0+e],[0 1 1 1-e 1-e 0+e],c3);plot([0 1 1 0 0],[0 0 1 1 0]);disp('b');
  netsum : Sum  Inputs=2  SaturateOnIntegerOverflow=on  InputSameDT=on  OutDataTypeStr=Inherit: Same as first input
      mask Display: e = 0.06;c1 = [120 150 230]/255;c2 = ([120 150 230]+0)/383;c3 = ([120 150 230]+128)/383;patch([0 1 1 0],[0 0 1 1],c1);patch([0 1 1 1-e 1-e 0+e],[0 0 1 1-e 0+e 0+e],c2);patch([0 0 1 1-e 0+e 0+e],[0 1 1 1-e 1-e 0+e],c3);plot([0 1 1 0 0],[0 0 1 1 0]);disp('+');
  purelin : neural/Transfer Functions/purelin
      settings: SourceProductName=Deep Learning Toolbox
  "a{7}" : Outport  InitialOutput=[0;0;0;0;0]
      mask Display: e = 0.06;c1 = [120 230 180]/255;c2 = ([120 230 180]+0)/(256+128);c3 = ([120 230 180]+128)/(256+128);patch([0 1 1 0],[0 0 1 1],c1);patch([0 1 1 1-e 1-e 0+e],[0 0 1 1-e 0+e 0+e],c2);patch([0 0 1 1-e 0+e 0+e],[0 1 1 1-e 1-e 0+e],c3);plot([0 1 1 0 0],[0 0 1 1 0]);
  wires:
  purelin/1 -> "a{7}"/1
  netsum/1 -> purelin/1
  "b{7}"/1 -> netsum/2
  "LW{7,6}"/1("lz{7,6}") -> netsum/1
  Delays 1/1("pd{7,6}") -> "LW{7,6}"/1("ad{7,6}")
  "a{6} "/1 -> Delays 1/1("p{7}")

== system AcasXuClosedLoop_all/networks/Networks_m_8/AcasXu_5_8/Process Input 1 ==
  x : Inport  PortDimensions=5
      mask Display: e = 0.06;c1 = [120 230 180]/255;c2 = ([120 230 180]+0)/(256+128);c3 = ([120 230 180]+128)/(256+128);patch([0 1 1 0],[0 0 1 1],c1);patch([0 1 1 1-e 1-e 0+e],[0 0 1 1-e 0+e 0+e],c2);patch([0 0 1 1-e 0+e 0+e],[0 1 1 1-e 1-e 0+e],c3);plot([0 1 1 0 0],[0 0 1 1 0]);
  p : Outport  PortDimensions=5
      mask Display: e = 0.06;c1 = [120 230 180]/255;c2 = ([120 230 180]+0)/(256+128);c3 = ([120 230 180]+128)/(256+128);patch([0 1 1 0],[0 0 1 1],c1);patch([0 1 1 1-e 1-e 0+e],[0 0 1 1-e 0+e 0+e],c2);patch([0 0 1 1-e 0+e 0+e],[0 1 1 1-e 1-e 0+e],c3);plot([0 1 1 0 0],[0 0 1 1 0]);
  wires:
  x/1 -> p/1

== system AcasXuClosedLoop_all/networks/Networks_m_8/AcasXu_5_8/Process Output 1 ==
  a : Inport  PortDimensions=5
      mask Display: e = 0.06;c1 = [120 230 180]/255;c2 = ([120 230 180]+0)/(256+128);c3 = ([120 230 180]+128)/(256+128);patch([0 1 1 0],[0 0 1 1],c1);patch([0 1 1 1-e 1-e 0+e],[0 0 1 1-e 0+e 0+e],c2);patch([0 0 1 1-e 0+e 0+e],[0 1 1 1-e 1-e 0+e],c3);plot([0 1 1 0 0],[0 0 1 1 0]);
  y : Outport  PortDimensions=5
      mask Display: e = 0.06;c1 = [120 230 180]/255;c2 = ([120 230 180]+0)/(256+128);c3 = ([120 230 180]+128)/(256+128);patch([0 1 1 0],[0 0 1 1],c1);patch([0 1 1 1-e 1-e 0+e],[0 0 1 1-e 0+e 0+e],c2);patch([0 0 1 1-e 0+e 0+e],[0 1 1 1-e 1-e 0+e],c3);plot([0 1 1 0 0],[0 0 1 1 0]);
  wires:
  a/1 -> y/1

== system AcasXuClosedLoop_all/networks/Networks_m_9/AcasXu_1_9/Layer 1 ==
  "p{1}" : Inport  PortDimensions=5
      mask Display: e = 0.06;c1 = [120 230 180]/255;c2 = ([120 230 180]+0)/(256+128);c3 = ([120 230 180]+128)/(256+128);patch([0 1 1 0],[0 0 1 1],c1);patch([0 1 1 1-e 1-e 0+e],[0 0 1 1-e 0+e 0+e],c2);patch([0 0 1 1-e 0+e 0+e],[0 1 1 1-e 1-e 0+e],c3);plot([0 1 1 0 0],[0 0 1 1 0]);
  Delays 1 : Subsystem
      mask Display: e = 0.06;c1 = [120 150 230]/255;c2 = ([120 150 230]+0)/383;c3 = ([120 150 230]+128)/383;patch([0 1 1 0],[0 0 1 1],c1);patch([0 1 1 1-e 1-e 0+e],[0 0 1 1-e 0+e 0+e],c2);patch([0 0 1 1-e 0+e 0+e],[0 1 1 1-e 1-e 0+e],c3);plot([0 1 1 0 0],[0 0 1 1 0]);disp('0');
  "IW{1,1}" : Subsystem
      mask Display: e = 0.06;c1 = [120 150 230]/255;c2 = ([120 150 230]+0)/383;c3 = ([120 150 230]+128)/383;patch([0 1 1 0],[0 0 1 1],c1);patch([0 1 1 1-e 1-e 0+e],[0 0 1 1-e 0+e 0+e],c2);patch([0 0 1 1-e 0+e 0+e],[0 1 1 1-e 1-e 0+e],c3);plot([0 1 1 0 0],[0 0 1 1 0]);disp('W');
  "b{1}" : Constant  Value=[0.2054479999999999917381643399494350887835025787353515625;-0.152932000000000012374101743262144736945629119873046875;-0.297323000000000003950617610826157033443450927734375;-0.07008989999999999664925809383930754847824573516845703125;-0.2304720000000000101891828307998366653919219970703125;0.02447749999999999925837101955039543099701404571533203125;-0.0623234000000000010199840971836238168179988861083984375;-0.01046260000000000071895822628675887244753539562225341796875;-0.2332700000000000051247894816697225905954837799072265625;-0.03815860000000000085140783312454004772007465362548828125;-0.1047680000000000000159872115546022541821002960205078125;-0.2408099999999999962785324214564752764999866485595703125;0.000814815999999999999482691581675908309989608824253082275390625;0.0571168999999999982275511456464300863444805145263671875;-0.308284000000000002472688720445148646831512451171875;0.1033020000000000049311665861750952899456024169921875;-0.0897029000000000020786927734661730937659740447998046875;-0.1101810000000000011599610161283635534346103668212890625;0.0149063000000000007994049866510977153666317462921142578125;0.07651529999999999442916731595687451772391796112060546875;0.076561299999999998799893319301190786063671112060546875;-0.000481030000000000020933532685063482858822681009769439697265625;-0.23624400000000000954258894125814549624919891357421875;0.0177100000000000000477395900588817312382161617279052734375;0.04963349999999999706545850131078623235225677490234375;0.051516499999999999792610339000020758248865604400634765625;0.0934228000000000002867039938792004249989986419677734375;-0.0831395000000000050643933491301140747964382171630859375;-0.0178515999999999987191134920294643961824476718902587890625;-0.1648340000000000082902573694809689186513423919677734375;-0.2495239999999999958024687884972081519663333892822265625;-0.124334000000000000074606987254810519516468048095703125;-0.1284579999999999888604662601210293360054492950439453125;0.0846317000000000041470826772638247348368167877197265625;0.0463440999999999991842969393474049866199493408203125;-0.00568896999999999979091835911049201968125998973846435546875;-0.016453399999999999969713115888225729577243328094482421875;0.21543699999999998961897063054493628442287445068359375;-0.1954829999999999901039160477012046612799167633056640625;0.068144700000000002493294459782191552221775054931640625;-0.2583480000000000220694573727087117731571197509765625;-0.002601550000000000216482387571659273817203938961029052734375;0.0235460000000000009567902026219599065370857715606689453125;-0.0087571400000000000629629681725418777205049991607666015625;0.057887399999999998578825710637829615734517574310302734375;-0.10223100000000000242561526420104200951755046844482421875;-0.0173824999999999986466381329819341772235929965972900390625;-0.01300970000000000077744477522401211899705231189727783203125;0.1750939999999999996393995616017491556704044342041015625;-0.07306179999999999619131330064192297868430614471435546875]
      mask Display: e = 0.06;c1 = [120 150 230]/255;c2 = ([120 150 230]+0)/383;c3 = ([120 150 230]+128)/383;patch([0 1 1 0],[0 0 1 1],c1);patch([0 1 1 1-e 1-e 0+e],[0 0 1 1-e 0+e 0+e],c2);patch([0 0 1 1-e 0+e 0+e],[0 1 1 1-e 1-e 0+e],c3);plot([0 1 1 0 0],[0 0 1 1 0]);disp('b');
  netsum : Sum  Inputs=2  SaturateOnIntegerOverflow=on  InputSameDT=on  OutDataTypeStr=Inherit: Same as first input
      mask Display: e = 0.06;c1 = [120 150 230]/255;c2 = ([120 150 230]+0)/383;c3 = ([120 150 230]+128)/383;patch([0 1 1 0],[0 0 1 1],c1);patch([0 1 1 1-e 1-e 0+e],[0 0 1 1-e 0+e 0+e],c2);patch([0 0 1 1-e 0+e 0+e],[0 1 1 1-e 1-e 0+e],c3);plot([0 1 1 0 0],[0 0 1 1 0]);disp('+');
  poslin : neural/Transfer Functions/poslin
      settings: SourceProductName=Deep Learning Toolbox  SourceProductBaseCode=NN
  "a{1}" : Outport  InitialOutput=[0;0;0;0;0;0;0;0;0;0;0;0;0;0;0;0;0;0;0;0;0;0;0;0;0;0;0;0;0;0;0;0;0;0;0;0;0;0;0;0;0;0;0;0;0;0;0;0;0;0]
      mask Display: e = 0.06;c1 = [120 230 180]/255;c2 = ([120 230 180]+0)/(256+128);c3 = ([120 230 180]+128)/(256+128);patch([0 1 1 0],[0 0 1 1],c1);patch([0 1 1 1-e 1-e 0+e],[0 0 1 1-e 0+e 0+e],c2);patch([0 0 1 1-e 0+e 0+e],[0 1 1 1-e 1-e 0+e],c3);plot([0 1 1 0 0],[0 0 1 1 0]);
  wires:
  poslin/1 -> "a{1}"/1
  netsum/1 -> poslin/1
  "b{1}"/1 -> netsum/2
  "IW{1,1}"/1("iz{1,1}") -> netsum/1
  Delays 1/1("pd{1,1}") -> "IW{1,1}"/1("pd{1,1}")
  "p{1}"/1 -> Delays 1/1("p{1}")

== system AcasXuClosedLoop_all/networks/Networks_m_9/AcasXu_1_9/Layer 2 ==
  "a{1} " : Inport  PortDimensions=50
      mask Display: e = 0.06;c1 = [120 230 180]/255;c2 = ([120 230 180]+0)/(256+128);c3 = ([120 230 180]+128)/(256+128);patch([0 1 1 0],[0 0 1 1],c1);patch([0 1 1 1-e 1-e 0+e],[0 0 1 1-e 0+e 0+e],c2);patch([0 0 1 1-e 0+e 0+e],[0 1 1 1-e 1-e 0+e],c3);plot([0 1 1 0 0],[0 0 1 1 0]);
  Delays 1 : Subsystem
      mask Display: e = 0.06;c1 = [120 150 230]/255;c2 = ([120 150 230]+0)/383;c3 = ([120 150 230]+128)/383;patch([0 1 1 0],[0 0 1 1],c1);patch([0 1 1 1-e 1-e 0+e],[0 0 1 1-e 0+e 0+e],c2);patch([0 0 1 1-e 0+e 0+e],[0 1 1 1-e 1-e 0+e],c3);plot([0 1 1 0 0],[0 0 1 1 0]);disp('0');
  "LW{2,1}" : Subsystem
      mask Display: e = 0.06;c1 = [120 150 230]/255;c2 = ([120 150 230]+0)/383;c3 = ([120 150 230]+128)/383;patch([0 1 1 0],[0 0 1 1],c1);patch([0 1 1 1-e 1-e 0+e],[0 0 1 1-e 0+e 0+e],c2);patch([0 0 1 1-e 0+e 0+e],[0 1 1 1-e 1-e 0+e],c3);plot([0 1 1 0 0],[0 0 1 1 0]);disp('W');
  "b{2}" : Constant  Value=[0.418217000000000005410782932813162915408611297607421875;0.10816399999999999625988067464277264662086963653564453125;0.0004284709999999999842541786865268704787013120949268341064453125;-0.0734898999999999968935071592568419873714447021484375;0.005813340000000000173940861714072525501251220703125;-0.55922799999999994735588870753417722880840301513671875;-0.2120990000000000097468699777891742996871471405029296875;-0.154947000000000001396216475768596865236759185791015625;0.03641350000000000142197364993990049697458744049072265625;0.140983999999999998209432305884547531604766845703125;-0.0218594000000000010519585202928283251821994781494140625;-0.064279699999999995174704281453159637749195098876953125;-0.06090790000000000092850171995451091788709163665771484375;-0.0275521999999999989139354283906868658959865570068359375;-0.0192412999999999993760990690816470305435359477996826171875;-0.09368200000000000138289379947309498675167560577392578125;0.0063350999999999997591704214983110432513058185577392578125;-0.01400069999999999960371699359029662446118891239166259765625;0.320782999999999984819254450485459528863430023193359375;-0.1787350000000000049826809345177025534212589263916015625;-0.020936799999999998467270501123493886552751064300537109375;-0.0282834999999999996134203428255204926244914531707763671875;1.043530000000000068638428274425677955150604248046875;-0.0642598000000000058040683370563783682882785797119140625;-0.0253698000000000013776091378758792416192591190338134765625;0.0216662000000000000421440660147709422744810581207275390625;-0.0044630499999999996563193604970365413464605808258056640625;-0.061781999999999996642241484323676559142768383026123046875;-0.008320660000000000555697710069580352865159511566162109375;-0.16217300000000001158895202024723403155803680419921875;-0.1410389999999999977031706066554761491715908050537109375;-0.016464499999999999968469666100645554251968860626220703125;0.005323099999999999880351264636146879638545215129852294921875;-0.08405899999999999483435431102407164871692657470703125;0.0265669000000000009309442106086862622760236263275146484375;0.07866910000000000569020386365082231350243091583251953125;-0.4908500000000000085265128291212022304534912109375;-0.0289568000000000012883472066960166557691991329193115234375;-0.01505309999999999981401543891479377634823322296142578125;-0.0074496600000000003094502432077206321991980075836181640625;-0.005955209999999999843922626752146243234165012836456298828125;0.09445639999999999592006361126550473272800445556640625;0.044334800000000000597122351564394193701446056365966796875;-0.0031892100000000000482869300100219334126450121402740478515625;-0.07632559999999999345021706176339648663997650146484375;0.048536999999999996813215830115950666368007659912109375;-0.31617499999999998383515276145772077143192291259765625;-0.36595699999999997675814711328712292015552520751953125;0.345880000000000020765611452588927932083606719970703125;0.057699699999999999600053257609033607877790927886962890625]
      mask Display: e = 0.06;c1 = [120 150 230]/255;c2 = ([120 150 230]+0)/383;c3 = ([120 150 230]+128)/383;patch([0 1 1 0],[0 0 1 1],c1);patch([0 1 1 1-e 1-e 0+e],[0 0 1 1-e 0+e 0+e],c2);patch([0 0 1 1-e 0+e 0+e],[0 1 1 1-e 1-e 0+e],c3);plot([0 1 1 0 0],[0 0 1 1 0]);disp('b');
  netsum : Sum  Inputs=2  SaturateOnIntegerOverflow=on  InputSameDT=on  OutDataTypeStr=Inherit: Same as first input
      mask Display: e = 0.06;c1 = [120 150 230]/255;c2 = ([120 150 230]+0)/383;c3 = ([120 150 230]+128)/383;patch([0 1 1 0],[0 0 1 1],c1);patch([0 1 1 1-e 1-e 0+e],[0 0 1 1-e 0+e 0+e],c2);patch([0 0 1 1-e 0+e 0+e],[0 1 1 1-e 1-e 0+e],c3);plot([0 1 1 0 0],[0 0 1 1 0]);disp('+');
  poslin : neural/Transfer Functions/poslin
      settings: SourceProductName=Deep Learning Toolbox  SourceProductBaseCode=NN
  "a{2}" : Outport  InitialOutput=[0;0;0;0;0;0;0;0;0;0;0;0;0;0;0;0;0;0;0;0;0;0;0;0;0;0;0;0;0;0;0;0;0;0;0;0;0;0;0;0;0;0;0;0;0;0;0;0;0;0]
      mask Display: e = 0.06;c1 = [120 230 180]/255;c2 = ([120 230 180]+0)/(256+128);c3 = ([120 230 180]+128)/(256+128);patch([0 1 1 0],[0 0 1 1],c1);patch([0 1 1 1-e 1-e 0+e],[0 0 1 1-e 0+e 0+e],c2);patch([0 0 1 1-e 0+e 0+e],[0 1 1 1-e 1-e 0+e],c3);plot([0 1 1 0 0],[0 0 1 1 0]);
  wires:
  poslin/1 -> "a{2}"/1
  netsum/1 -> poslin/1
  "b{2}"/1 -> netsum/2
  "LW{2,1}"/1("lz{2,1}") -> netsum/1
  Delays 1/1("pd{2,1}") -> "LW{2,1}"/1("ad{2,1}")
  "a{1} "/1 -> Delays 1/1("p{2}")
